simulink subsystem "ButtonStatesRF"  (R2023b) (continued)
== stateflow chart ButtonStatesRF/ButtonStates/buttonFlowChart == (continued)
                  Flow.ButtonTriggeredMenu.Menu.MainSubMenu.SettingsSubMenu.FavoritesSubMenu.Slot1Menu.Slot1FavoritesMenu.Slot1Date -> Flow.ButtonTriggeredMenu.Menu.MainSubMenu.SettingsSubMenu.FavoritesSubMenu.Slot1Menu.Slot1FavoritesMenu.Slot1Battery [order 2] : DOWN
                  Flow.ButtonTriggeredMenu.Menu.MainSubMenu.SettingsSubMenu.FavoritesSubMenu.Slot1Menu.Slot1FavoritesMenu.Slot1Battery -> Flow.ButtonTriggeredMenu.Menu.MainSubMenu.SettingsSubMenu.FavoritesSubMenu.Slot1Menu.Slot1FavoritesMenu.Slot1Date [order 1] : UP
                  Flow.ButtonTriggeredMenu.Menu.MainSubMenu.SettingsSubMenu.FavoritesSubMenu.Slot1Menu.Slot1FavoritesMenu.Slot1Battery -> Flow.ButtonTriggeredMenu.Menu.MainSubMenu.SettingsSubMenu.FavoritesSubMenu.Slot1Menu.Slot1FavoritesMenu.Slot1WaterTemperature [order 2] : DOWN
                  Flow.ButtonTriggeredMenu.Menu.MainSubMenu.SettingsSubMenu.FavoritesSubMenu.Slot1Menu.Slot1FavoritesMenu.Slot1WaterTemperature -> Flow.ButtonTriggeredMenu.Menu.MainSubMenu.SettingsSubMenu.FavoritesSubMenu.Slot1Menu.Slot1FavoritesMenu.Slot1Battery [order 1] : UP
                  Flow.ButtonTriggeredMenu.Menu.MainSubMenu.SettingsSubMenu.FavoritesSubMenu.Slot1Menu.Slot1FavoritesMenu.Slot1WaterTemperature -> Flow.ButtonTriggeredMenu.Menu.MainSubMenu.SettingsSubMenu.FavoritesSubMenu.Slot1Menu.Slot1FavoritesMenu.Slot1Odometer [order 2] : DOWN
                  Flow.ButtonTriggeredMenu.Menu.MainSubMenu.SettingsSubMenu.FavoritesSubMenu.Slot1Menu.Slot1FavoritesMenu.Slot1Odometer -> Flow.ButtonTriggeredMenu.Menu.MainSubMenu.SettingsSubMenu.FavoritesSubMenu.Slot1Menu.Slot1FavoritesMenu.Slot1WaterTemperature [order 1] : UP
                  Flow.ButtonTriggeredMenu.Menu.MainSubMenu.SettingsSubMenu.FavoritesSubMenu.Slot1Menu.Slot1FavoritesMenu.Slot1Odometer -> Flow.ButtonTriggeredMenu.Menu.MainSubMenu.SettingsSubMenu.FavoritesSubMenu.Slot1Menu.Slot1FavoritesMenu.Slot1Connectivity [order 2] : DOWN
                  Flow.ButtonTriggeredMenu.Menu.MainSubMenu.SettingsSubMenu.FavoritesSubMenu.Slot1Menu.Slot1FavoritesMenu.Slot1Connectivity -> Flow.ButtonTriggeredMenu.Menu.MainSubMenu.SettingsSubMenu.FavoritesSubMenu.Slot1Menu.Slot1FavoritesMenu.Slot1Odometer [order 1] : UP
                  (junction 6434) -> Flow.ButtonTriggeredMenu.Menu.MainSubMenu.SettingsSubMenu.FavoritesSubMenu.Slot1Menu.Slot1FavoritesMenu.Slot1Connectivity
                  Flow.ButtonTriggeredMenu.Menu.MainSubMenu.SettingsSubMenu.FavoritesSubMenu.Slot1Menu.Slot1FavoritesMenu.Slot1Connectivity -> (junction 6435) [order 2] : DOWN
                state Slot1FavoritesMenu1 [commented out]
                  state Slot1TripDistance1 [commented out]
                    en:
                    currentState = eStateName.FAVORITES_TRIP_DISTANCE_1;
                  state Slot1AverageSpeed1 [commented out]
                    en:
                    currentState = eStateName.FAVORITES_AVERAGE_SPEED_1;
                  state Slot1AverageFuelCons1 [commented out]
                    en:
                    currentState = eStateName.FAVORITES_AVERAGE_FUEL_CONS_1;
                  state Slot1TripTime1 [commented out]
                    en:
                    currentState = eStateName.FAVORITES_TRIP_TIME_1;
                  state Slot1AverageTripDistance2 [commented out]
                    en:
                    currentState = eStateName.FAVORITES_TRIP_DISTANCE_2;
                  state Slot1AverageSpeed2 [commented out]
                    en:
                    currentState = eStateName.FAVORITES_AVERAGE_SPEED_2;
                  state Slot1AverageFuelCons2 [commented out]
                    en:
                    currentState = eStateName.FAVORITES_AVERAGE_FUEL_CONS_2;
                  state Slot1TripTime2 [commented out]
                    en:
                    currentState = eStateName.FAVORITES_TRIP_TIME_2;
                  state Slot1FuelRange [commented out]
                    en:
                    currentState = eStateName.FAVORITES_FUEL_RANGE;
                  state Slot1Date [commented out]
                    en:
                    currentState = eStateName.FAVORITES_DATE;
                  state Slot1Battery [commented out]
                    en:
                    currentState = eStateName.FAVORITES_BATTERY;
                  state Slot1WaterTemperature [commented out]
                    en:
                    currentState = eStateName.FAVORITES_WATER_TEMPERATURE;
                  state Slot1Odometer [commented out]
                    en:
                    currentState = eStateName.FAVORITES_ODOMETER;
                  state Slot1Connectivity [commented out]
                    en:
                    currentState = eStateName.FAVORITES_CONNECTIVITY;
                  8721 -> (junction 8703) [commented out]
                  (default) -> (junction 8703) [commented out]
                  (junction 8703) -> (junction 8704) [commented out] : [favSlot1Sel ==eStateName.FAVORITES_TRIP_DISTANCE_1 ]
                  (junction 8704) -> Flow.ButtonTriggeredMenu.Menu.MainSubMenu.SettingsSubMenu.FavoritesSubMenu.Slot1Menu.Slot1FavoritesMenu1.Slot1TripDistance1 [commented out]
                  Flow.ButtonTriggeredMenu.Menu.MainSubMenu.SettingsSubMenu.FavoritesSubMenu.Slot1Menu.Slot1FavoritesMenu1.Slot1TripDistance1 -> (junction 8705) [commented out] : UP
                  (junction 8703) -> (junction 8706) [commented out]
                  (junction 8720) -> (junction 8704) [commented out]
                  (junction 8705) -> (junction 8719) [commented out]
                  Flow.ButtonTriggeredMenu.Menu.MainSubMenu.SettingsSubMenu.FavoritesSubMenu.Slot1Menu.Slot1FavoritesMenu1.Slot1TripDistance1 -> Flow.ButtonTriggeredMenu.Menu.MainSubMenu.SettingsSubMenu.FavoritesSubMenu.Slot1Menu.Slot1FavoritesMenu1.Slot1AverageSpeed1 [commented out] : DOWN
                  Flow.ButtonTriggeredMenu.Menu.MainSubMenu.SettingsSubMenu.FavoritesSubMenu.Slot1Menu.Slot1FavoritesMenu1.Slot1AverageSpeed1 -> Flow.ButtonTriggeredMenu.Menu.MainSubMenu.SettingsSubMenu.FavoritesSubMenu.Slot1Menu.Slot1FavoritesMenu1.Slot1TripDistance1 [commented out] : UP
                  (junction 8706) -> Flow.ButtonTriggeredMenu.Menu.MainSubMenu.SettingsSubMenu.FavoritesSubMenu.Slot1Menu.Slot1FavoritesMenu1.Slot1AverageSpeed1 [commented out] : [favSlot1Sel ==eStateName.FAVORITES_AVERAGE_SPEED_1 ]
                  (junction 8706) -> (junction 8707) [commented out]
                  Flow.ButtonTriggeredMenu.Menu.MainSubMenu.SettingsSubMenu.FavoritesSubMenu.Slot1Menu.Slot1FavoritesMenu1.Slot1AverageSpeed1 -> Flow.ButtonTriggeredMenu.Menu.MainSubMenu.SettingsSubMenu.FavoritesSubMenu.Slot1Menu.Slot1FavoritesMenu1.Slot1AverageFuelCons1 [commented out] : DOWN
                  Flow.ButtonTriggeredMenu.Menu.MainSubMenu.SettingsSubMenu.FavoritesSubMenu.Slot1Menu.Slot1FavoritesMenu1.Slot1AverageFuelCons1 -> Flow.ButtonTriggeredMenu.Menu.MainSubMenu.SettingsSubMenu.FavoritesSubMenu.Slot1Menu.Slot1FavoritesMenu1.Slot1AverageSpeed1 [commented out] : UP
                  (junction 8707) -> Flow.ButtonTriggeredMenu.Menu.MainSubMenu.SettingsSubMenu.FavoritesSubMenu.Slot1Menu.Slot1FavoritesMenu1.Slot1AverageFuelCons1 [commented out] : [favSlot1Sel ==eStateName.FAVORITES_AVERAGE_FUEL_CONS_1 ]
                  (junction 8707) -> (junction 8708) [commented out]
                  Flow.ButtonTriggeredMenu.Menu.MainSubMenu.SettingsSubMenu.FavoritesSubMenu.Slot1Menu.Slot1FavoritesMenu1.Slot1AverageFuelCons1 -> Flow.ButtonTriggeredMenu.Menu.MainSubMenu.SettingsSubMenu.FavoritesSubMenu.Slot1Menu.Slot1FavoritesMenu1.Slot1TripTime1 [commented out] : DOWN
                  Flow.ButtonTriggeredMenu.Menu.MainSubMenu.SettingsSubMenu.FavoritesSubMenu.Slot1Menu.Slot1FavoritesMenu1.Slot1TripTime1 -> Flow.ButtonTriggeredMenu.Menu.MainSubMenu.SettingsSubMenu.FavoritesSubMenu.Slot1Menu.Slot1FavoritesMenu1.Slot1AverageFuelCons1 [commented out] : UP
                  (junction 8708) -> Flow.ButtonTriggeredMenu.Menu.MainSubMenu.SettingsSubMenu.FavoritesSubMenu.Slot1Menu.Slot1FavoritesMenu1.Slot1TripTime1 [commented out] : [favSlot1Sel ==eStateName.FAVORITES_TRIP_TIME_1 ]
                  (junction 8708) -> (junction 8709) [commented out]
                  Flow.ButtonTriggeredMenu.Menu.MainSubMenu.SettingsSubMenu.FavoritesSubMenu.Slot1Menu.Slot1FavoritesMenu1.Slot1TripTime1 -> Flow.ButtonTriggeredMenu.Menu.MainSubMenu.SettingsSubMenu.FavoritesSubMenu.Slot1Menu.Slot1FavoritesMenu1.Slot1AverageTripDistance2 [commented out] : DOWN
                  Flow.ButtonTriggeredMenu.Menu.MainSubMenu.SettingsSubMenu.FavoritesSubMenu.Slot1Menu.Slot1FavoritesMenu1.Slot1AverageTripDistance2 -> Flow.ButtonTriggeredMenu.Menu.MainSubMenu.SettingsSubMenu.FavoritesSubMenu.Slot1Menu.Slot1FavoritesMenu1.Slot1TripTime1 [commented out] : UP
                  (junction 8709) -> Flow.ButtonTriggeredMenu.Menu.MainSubMenu.SettingsSubMenu.FavoritesSubMenu.Slot1Menu.Slot1FavoritesMenu1.Slot1AverageTripDistance2 [commented out] : [favSlot1Sel ==eStateName.FAVORITES_TRIP_DISTANCE_2 ]
                  (junction 8709) -> (junction 8710) [commented out]
                  Flow.ButtonTriggeredMenu.Menu.MainSubMenu.SettingsSubMenu.FavoritesSubMenu.Slot1Menu.Slot1FavoritesMenu1.Slot1AverageTripDistance2 -> Flow.ButtonTriggeredMenu.Menu.MainSubMenu.SettingsSubMenu.FavoritesSubMenu.Slot1Menu.Slot1FavoritesMenu1.Slot1AverageSpeed2 [commented out] : DOWN
                  Flow.ButtonTriggeredMenu.Menu.MainSubMenu.SettingsSubMenu.FavoritesSubMenu.Slot1Menu.Slot1FavoritesMenu1.Slot1AverageSpeed2 -> Flow.ButtonTriggeredMenu.Menu.MainSubMenu.SettingsSubMenu.FavoritesSubMenu.Slot1Menu.Slot1FavoritesMenu1.Slot1AverageTripDistance2 [commented out] : UP
                  (junction 8710) -> Flow.ButtonTriggeredMenu.Menu.MainSubMenu.SettingsSubMenu.FavoritesSubMenu.Slot1Menu.Slot1FavoritesMenu1.Slot1AverageSpeed2 [commented out] : [favSlot1Sel ==eStateName.FAVORITES_AVERAGE_SPEED_2 ]
                  (junction 8710) -> (junction 8711) [commented out]
                  Flow.ButtonTriggeredMenu.Menu.MainSubMenu.SettingsSubMenu.FavoritesSubMenu.Slot1Menu.Slot1FavoritesMenu1.Slot1AverageSpeed2 -> Flow.ButtonTriggeredMenu.Menu.MainSubMenu.SettingsSubMenu.FavoritesSubMenu.Slot1Menu.Slot1FavoritesMenu1.Slot1AverageFuelCons2 [commented out] : DOWN
                  Flow.ButtonTriggeredMenu.Menu.MainSubMenu.SettingsSubMenu.FavoritesSubMenu.Slot1Menu.Slot1FavoritesMenu1.Slot1AverageFuelCons2 -> Flow.ButtonTriggeredMenu.Menu.MainSubMenu.SettingsSubMenu.FavoritesSubMenu.Slot1Menu.Slot1FavoritesMenu1.Slot1AverageSpeed2 [commented out] : UP
                  (junction 8711) -> Flow.ButtonTriggeredMenu.Menu.MainSubMenu.SettingsSubMenu.FavoritesSubMenu.Slot1Menu.Slot1FavoritesMenu1.Slot1AverageFuelCons2 [commented out] : [favSlot1Sel ==eStateName.FAVORITES_AVERAGE_FUEL_CONS_2 ]
                  (junction 8711) -> (junction 8712) [commented out]
                  Flow.ButtonTriggeredMenu.Menu.MainSubMenu.SettingsSubMenu.FavoritesSubMenu.Slot1Menu.Slot1FavoritesMenu1.Slot1AverageFuelCons2 -> Flow.ButtonTriggeredMenu.Menu.MainSubMenu.SettingsSubMenu.FavoritesSubMenu.Slot1Menu.Slot1FavoritesMenu1.Slot1TripTime2 [commented out] : DOWN
                  Flow.ButtonTriggeredMenu.Menu.MainSubMenu.SettingsSubMenu.FavoritesSubMenu.Slot1Menu.Slot1FavoritesMenu1.Slot1TripTime2 -> Flow.ButtonTriggeredMenu.Menu.MainSubMenu.SettingsSubMenu.FavoritesSubMenu.Slot1Menu.Slot1FavoritesMenu1.Slot1AverageFuelCons2 [commented out] : UP
                  (junction 8712) -> Flow.ButtonTriggeredMenu.Menu.MainSubMenu.SettingsSubMenu.FavoritesSubMenu.Slot1Menu.Slot1FavoritesMenu1.Slot1TripTime2 [commented out] : [favSlot1Sel ==eStateName.FAVORITES_TRIP_TIME_2 ]
                  (junction 8712) -> (junction 8713) [commented out]
                  Flow.ButtonTriggeredMenu.Menu.MainSubMenu.SettingsSubMenu.FavoritesSubMenu.Slot1Menu.Slot1FavoritesMenu1.Slot1TripTime2 -> Flow.ButtonTriggeredMenu.Menu.MainSubMenu.SettingsSubMenu.FavoritesSubMenu.Slot1Menu.Slot1FavoritesMenu1.Slot1FuelRange [commented out] : DOWN
                  Flow.ButtonTriggeredMenu.Menu.MainSubMenu.SettingsSubMenu.FavoritesSubMenu.Slot1Menu.Slot1FavoritesMenu1.Slot1FuelRange -> Flow.ButtonTriggeredMenu.Menu.MainSubMenu.SettingsSubMenu.FavoritesSubMenu.Slot1Menu.Slot1FavoritesMenu1.Slot1TripTime2 [commented out] : UP
                  (junction 8713) -> Flow.ButtonTriggeredMenu.Menu.MainSubMenu.SettingsSubMenu.FavoritesSubMenu.Slot1Menu.Slot1FavoritesMenu1.Slot1FuelRange [commented out] : [favSlot1Sel ==eStateName.FAVORITES_FUEL_RANGE ]
                  (junction 8713) -> (junction 8714) [commented out]
                  Flow.ButtonTriggeredMenu.Menu.MainSubMenu.SettingsSubMenu.FavoritesSubMenu.Slot1Menu.Slot1FavoritesMenu1.Slot1FuelRange -> Flow.ButtonTriggeredMenu.Menu.MainSubMenu.SettingsSubMenu.FavoritesSubMenu.Slot1Menu.Slot1FavoritesMenu1.Slot1Date [commented out] : DOWN
                  Flow.ButtonTriggeredMenu.Menu.MainSubMenu.SettingsSubMenu.FavoritesSubMenu.Slot1Menu.Slot1FavoritesMenu1.Slot1Date -> Flow.ButtonTriggeredMenu.Menu.MainSubMenu.SettingsSubMenu.FavoritesSubMenu.Slot1Menu.Slot1FavoritesMenu1.Slot1FuelRange [commented out] : UP
                  (junction 8714) -> Flow.ButtonTriggeredMenu.Menu.MainSubMenu.SettingsSubMenu.FavoritesSubMenu.Slot1Menu.Slot1FavoritesMenu1.Slot1Date [commented out] : [favSlot1Sel ==eStateName.FAVORITES_DATE ]
                  (junction 8714) -> (junction 8715) [commented out]
                  Flow.ButtonTriggeredMenu.Menu.MainSubMenu.SettingsSubMenu.FavoritesSubMenu.Slot1Menu.Slot1FavoritesMenu1.Slot1Date -> Flow.ButtonTriggeredMenu.Menu.MainSubMenu.SettingsSubMenu.FavoritesSubMenu.Slot1Menu.Slot1FavoritesMenu1.Slot1Battery [commented out] : DOWN
                  Flow.ButtonTriggeredMenu.Menu.MainSubMenu.SettingsSubMenu.FavoritesSubMenu.Slot1Menu.Slot1FavoritesMenu1.Slot1Battery -> Flow.ButtonTriggeredMenu.Menu.MainSubMenu.SettingsSubMenu.FavoritesSubMenu.Slot1Menu.Slot1FavoritesMenu1.Slot1Date [commented out] : UP
                  (junction 8715) -> Flow.ButtonTriggeredMenu.Menu.MainSubMenu.SettingsSubMenu.FavoritesSubMenu.Slot1Menu.Slot1FavoritesMenu1.Slot1Battery [commented out] : [favSlot1Sel ==eStateName.FAVORITES_BATTERY ]
                  (junction 8715) -> (junction 8716) [commented out]
                  Flow.ButtonTriggeredMenu.Menu.MainSubMenu.SettingsSubMenu.FavoritesSubMenu.Slot1Menu.Slot1FavoritesMenu1.Slot1Battery -> Flow.ButtonTriggeredMenu.Menu.MainSubMenu.SettingsSubMenu.FavoritesSubMenu.Slot1Menu.Slot1FavoritesMenu1.Slot1WaterTemperature [commented out] : DOWN
                  Flow.ButtonTriggeredMenu.Menu.MainSubMenu.SettingsSubMenu.FavoritesSubMenu.Slot1Menu.Slot1FavoritesMenu1.Slot1WaterTemperature -> Flow.ButtonTriggeredMenu.Menu.MainSubMenu.SettingsSubMenu.FavoritesSubMenu.Slot1Menu.Slot1FavoritesMenu1.Slot1Battery [commented out] : UP
                  (junction 8716) -> Flow.ButtonTriggeredMenu.Menu.MainSubMenu.SettingsSubMenu.FavoritesSubMenu.Slot1Menu.Slot1FavoritesMenu1.Slot1WaterTemperature [commented out] : [favSlot1Sel ==eStateName.FAVORITES_WATER_TEMPERATURE ]
                  (junction 8716) -> (junction 8717) [commented out]
                  Flow.ButtonTriggeredMenu.Menu.MainSubMenu.SettingsSubMenu.FavoritesSubMenu.Slot1Menu.Slot1FavoritesMenu1.Slot1WaterTemperature -> Flow.ButtonTriggeredMenu.Menu.MainSubMenu.SettingsSubMenu.FavoritesSubMenu.Slot1Menu.Slot1FavoritesMenu1.Slot1Odometer [commented out] : DOWN
                  Flow.ButtonTriggeredMenu.Menu.MainSubMenu.SettingsSubMenu.FavoritesSubMenu.Slot1Menu.Slot1FavoritesMenu1.Slot1Odometer -> Flow.ButtonTriggeredMenu.Menu.MainSubMenu.SettingsSubMenu.FavoritesSubMenu.Slot1Menu.Slot1FavoritesMenu1.Slot1WaterTemperature [commented out] : UP
                  (junction 8717) -> Flow.ButtonTriggeredMenu.Menu.MainSubMenu.SettingsSubMenu.FavoritesSubMenu.Slot1Menu.Slot1FavoritesMenu1.Slot1Odometer [commented out] : [favSlot1Sel ==eStateName.FAVORITES_ODOMETER ]
                  (junction 8717) -> (junction 8718) [commented out]
                  Flow.ButtonTriggeredMenu.Menu.MainSubMenu.SettingsSubMenu.FavoritesSubMenu.Slot1Menu.Slot1FavoritesMenu1.Slot1Odometer -> Flow.ButtonTriggeredMenu.Menu.MainSubMenu.SettingsSubMenu.FavoritesSubMenu.Slot1Menu.Slot1FavoritesMenu1.Slot1Connectivity [commented out] : DOWN
                  Flow.ButtonTriggeredMenu.Menu.MainSubMenu.SettingsSubMenu.FavoritesSubMenu.Slot1Menu.Slot1FavoritesMenu1.Slot1Connectivity -> Flow.ButtonTriggeredMenu.Menu.MainSubMenu.SettingsSubMenu.FavoritesSubMenu.Slot1Menu.Slot1FavoritesMenu1.Slot1Odometer [commented out] : UP
                  (junction 8718) -> Flow.ButtonTriggeredMenu.Menu.MainSubMenu.SettingsSubMenu.FavoritesSubMenu.Slot1Menu.Slot1FavoritesMenu1.Slot1Connectivity [commented out]
                  (junction 8719) -> Flow.ButtonTriggeredMenu.Menu.MainSubMenu.SettingsSubMenu.FavoritesSubMenu.Slot1Menu.Slot1FavoritesMenu1.Slot1Connectivity [commented out]
                  Flow.ButtonTriggeredMenu.Menu.MainSubMenu.SettingsSubMenu.FavoritesSubMenu.Slot1Menu.Slot1FavoritesMenu1.Slot1Connectivity -> (junction 8720) [commented out] : DOWN
                state favSlot1Updater(currState) [function]
                  (default) -> (junction 6485) : %{tempSlotSel  = eStateName.FAVORITES_TRIP_DISTANCE_1;}
                  (junction 6485) -> (junction 6486) [order 1] : [currState == favSlot2Sel ]
                  (junction 6486) -> (junction 6487) : { favSlot2Sel = favSlot1Sel;
                      | BLU_FnSaveSlot2Fav(favSlot2Sel);}
                  (junction 6485) -> (junction 6488) [order 2]
                  (junction 6487) -> (junction 6490)
                  (junction 6488) -> (junction 6489) [order 1] : [currState == favSlot3Sel]
                  (junction 6489) -> (junction 6490) : {favSlot3Sel = favSlot1Sel;
                      | BLU_FnSaveSlot3Fav(favSlot3Sel);}
                  (junction 6488) -> (junction 6491) [order 2]
                  (junction 6490) -> (junction 6493)
                  (junction 6491) -> (junction 6492) [order 1] : [currState == favSlot4Sel ]
                  (junction 6492) -> (junction 6493) : {favSlot4Sel = favSlot1Sel;
                      | BLU_FnSaveSlot4Fav(favSlot4Sel);}
                  (junction 6491) -> (junction 6494) [order 2]
                  (junction 6493) -> (junction 6495)
                  (junction 6495) -> (junction 6494)
                  (junction 6494) -> (junction 6496) : {favSlot1Sel = currState;
                      | BLU_FnSaveSlot1Fav(favSlot1Sel);}
                state favSlotUpdater(currState) [function] [commented out]
                  (default) -> (junction 8448) [commented out] : %{tempSlotSel  = eStateName.FAVORITES_TRIP_DISTANCE_1;}
                  (junction 8448) -> (junction 8449) [commented out] : [currState == favSlot2Sel ]
                  (junction 8449) -> (junction 8450) [commented out] : { favSlot2Sel = favSlot1Sel;
                      | BLU_FnSaveSlot2Fav(favSlot2Sel);}
                  (junction 8448) -> (junction 8451) [commented out]
                  (junction 8450) -> (junction 8453) [commented out]
                  (junction 8451) -> (junction 8452) [commented out] : [currState == favSlot3Sel]
                  (junction 8452) -> (junction 8453) [commented out] : {favSlot3Sel = favSlot1Sel;
                      | BLU_FnSaveSlot3Fav(favSlot3Sel);}
                  (junction 8451) -> (junction 8454) [commented out]
                  (junction 8453) -> (junction 8456) [commented out]
                  (junction 8454) -> (junction 8455) [commented out] : [currState == favSlot4Sel ]
                  (junction 8455) -> (junction 8456) [commented out] : {favSlot4Sel = favSlot1Sel;
                      | BLU_FnSaveSlot4Fav(favSlot4Sel);}
                  (junction 8454) -> (junction 8457) [commented out]
                  (junction 8456) -> (junction 8458) [commented out]
                  (junction 8458) -> (junction 8457) [commented out]
                  (junction 8457) -> (junction 8459) [commented out] : {favSlot1Sel = currState;
                      | BLU_FnSaveSlot1Fav(favSlot1Sel);}
                6424 -> Slot1FavoritesMenu
                Slot1FavoritesMenu -> 6425 [order 2] : EXIT
                (junction 6423) -> 6425 : {favSlot1Updater(currentState);}
                Slot1FavoritesMenu -> (junction 6423) [order 1] : SET
              state Slot2Menu
                state Slot2FavoritesMenu
                  (history)
                  state Slot2TripDistance1
                    en:
                    currentState = eStateName.FAVORITES_TRIP_DISTANCE_1;
                  state Slot2AverageSpeed1
                    en:
                    currentState = eStateName.FAVORITES_AVERAGE_SPEED_1;
                  state Slot2AverageFuelCons1
                    en:
                    currentState = eStateName.FAVORITES_AVERAGE_FUEL_CONS_1;
                  state Slot2TripTime1
                    en:
                    currentState = eStateName.FAVORITES_TRIP_TIME_1;
                  state Slot2AverageTripDistance2
                    en:
                    currentState = eStateName.FAVORITES_TRIP_DISTANCE_2;
                  state Slot2AverageSpeed2
                    en:
                    currentState = eStateName.FAVORITES_AVERAGE_SPEED_2;
                  state Slot2AverageFuelCons2
                    en:
                    currentState = eStateName.FAVORITES_AVERAGE_FUEL_CONS_2;
                  state Slot2TripTime2
                    en:
                    currentState = eStateName.FAVORITES_TRIP_TIME_2;
                  state Slot2FuelRange
                    en:
                    currentState = eStateName.FAVORITES_FUEL_RANGE;
                  state Slot2Date
                    en:
                    currentState = eStateName.FAVORITES_DATE;
                  state Slot2Battery
                    en:
                    currentState = eStateName.FAVORITES_BATTERY;
                  state Slot2WaterTemperature
                    en:
                    currentState = eStateName.FAVORITES_WATER_TEMPERATURE;
                  state Slot2Odometer
                    en:
                    currentState = eStateName.FAVORITES_ODOMETER;
                  state Slot2Connectivity
                    en:
                    currentState = eStateName.FAVORITES_CONNECTIVITY;
                  (default) -> (junction 6523)
                  Flow.ButtonTriggeredMenu.Menu.MainSubMenu.SettingsSubMenu.FavoritesSubMenu.Slot2Menu.Slot2FavoritesMenu.Slot2TripDistance1 -> (junction 6522) [order 2] : UP
                  (junction 6523) -> Flow.ButtonTriggeredMenu.Menu.MainSubMenu.SettingsSubMenu.FavoritesSubMenu.Slot2Menu.Slot2FavoritesMenu.Slot2TripDistance1
                  (junction 6522) -> (junction 6525)
                  (junction 6524) -> (junction 6523)
                  Flow.ButtonTriggeredMenu.Menu.MainSubMenu.SettingsSubMenu.FavoritesSubMenu.Slot2Menu.Slot2FavoritesMenu.Slot2TripDistance1 -> Flow.ButtonTriggeredMenu.Menu.MainSubMenu.SettingsSubMenu.FavoritesSubMenu.Slot2Menu.Slot2FavoritesMenu.Slot2AverageSpeed1 [order 1] : DOWN
                  Flow.ButtonTriggeredMenu.Menu.MainSubMenu.SettingsSubMenu.FavoritesSubMenu.Slot2Menu.Slot2FavoritesMenu.Slot2AverageSpeed1 -> Flow.ButtonTriggeredMenu.Menu.MainSubMenu.SettingsSubMenu.FavoritesSubMenu.Slot2Menu.Slot2FavoritesMenu.Slot2TripDistance1 [order 1] : UP
                  Flow.ButtonTriggeredMenu.Menu.MainSubMenu.SettingsSubMenu.FavoritesSubMenu.Slot2Menu.Slot2FavoritesMenu.Slot2AverageSpeed1 -> Flow.ButtonTriggeredMenu.Menu.MainSubMenu.SettingsSubMenu.FavoritesSubMenu.Slot2Menu.Slot2FavoritesMenu.Slot2AverageFuelCons1 [order 2] : DOWN
                  Flow.ButtonTriggeredMenu.Menu.MainSubMenu.SettingsSubMenu.FavoritesSubMenu.Slot2Menu.Slot2FavoritesMenu.Slot2AverageFuelCons1 -> Flow.ButtonTriggeredMenu.Menu.MainSubMenu.SettingsSubMenu.FavoritesSubMenu.Slot2Menu.Slot2FavoritesMenu.Slot2AverageSpeed1 [order 1] : UP
                  Flow.ButtonTriggeredMenu.Menu.MainSubMenu.SettingsSubMenu.FavoritesSubMenu.Slot2Menu.Slot2FavoritesMenu.Slot2AverageFuelCons1 -> Flow.ButtonTriggeredMenu.Menu.MainSubMenu.SettingsSubMenu.FavoritesSubMenu.Slot2Menu.Slot2FavoritesMenu.Slot2TripTime1 [order 2] : DOWN
                  Flow.ButtonTriggeredMenu.Menu.MainSubMenu.SettingsSubMenu.FavoritesSubMenu.Slot2Menu.Slot2FavoritesMenu.Slot2TripTime1 -> Flow.ButtonTriggeredMenu.Menu.MainSubMenu.SettingsSubMenu.FavoritesSubMenu.Slot2Menu.Slot2FavoritesMenu.Slot2AverageFuelCons1 [order 1] : UP
                  Flow.ButtonTriggeredMenu.Menu.MainSubMenu.SettingsSubMenu.FavoritesSubMenu.Slot2Menu.Slot2FavoritesMenu.Slot2TripTime1 -> Flow.ButtonTriggeredMenu.Menu.MainSubMenu.SettingsSubMenu.FavoritesSubMenu.Slot2Menu.Slot2FavoritesMenu.Slot2AverageTripDistance2 [order 2] : DOWN
                  Flow.ButtonTriggeredMenu.Menu.MainSubMenu.SettingsSubMenu.FavoritesSubMenu.Slot2Menu.Slot2FavoritesMenu.Slot2AverageTripDistance2 -> Flow.ButtonTriggeredMenu.Menu.MainSubMenu.SettingsSubMenu.FavoritesSubMenu.Slot2Menu.Slot2FavoritesMenu.Slot2TripTime1 [order 1] : UP
                  Flow.ButtonTriggeredMenu.Menu.MainSubMenu.SettingsSubMenu.FavoritesSubMenu.Slot2Menu.Slot2FavoritesMenu.Slot2AverageTripDistance2 -> Flow.ButtonTriggeredMenu.Menu.MainSubMenu.SettingsSubMenu.FavoritesSubMenu.Slot2Menu.Slot2FavoritesMenu.Slot2AverageSpeed2 [order 2] : DOWN
                  Flow.ButtonTriggeredMenu.Menu.MainSubMenu.SettingsSubMenu.FavoritesSubMenu.Slot2Menu.Slot2FavoritesMenu.Slot2AverageSpeed2 -> Flow.ButtonTriggeredMenu.Menu.MainSubMenu.SettingsSubMenu.FavoritesSubMenu.Slot2Menu.Slot2FavoritesMenu.Slot2AverageTripDistance2 [order 1] : UP
                  Flow.ButtonTriggeredMenu.Menu.MainSubMenu.SettingsSubMenu.FavoritesSubMenu.Slot2Menu.Slot2FavoritesMenu.Slot2AverageSpeed2 -> Flow.ButtonTriggeredMenu.Menu.MainSubMenu.SettingsSubMenu.FavoritesSubMenu.Slot2Menu.Slot2FavoritesMenu.Slot2AverageFuelCons2 [order 2] : DOWN
                  Flow.ButtonTriggeredMenu.Menu.MainSubMenu.SettingsSubMenu.FavoritesSubMenu.Slot2Menu.Slot2FavoritesMenu.Slot2AverageFuelCons2 -> Flow.ButtonTriggeredMenu.Menu.MainSubMenu.SettingsSubMenu.FavoritesSubMenu.Slot2Menu.Slot2FavoritesMenu.Slot2AverageSpeed2 [order 1] : UP
                  Flow.ButtonTriggeredMenu.Menu.MainSubMenu.SettingsSubMenu.FavoritesSubMenu.Slot2Menu.Slot2FavoritesMenu.Slot2AverageFuelCons2 -> Flow.ButtonTriggeredMenu.Menu.MainSubMenu.SettingsSubMenu.FavoritesSubMenu.Slot2Menu.Slot2FavoritesMenu.Slot2TripTime2 [order 2] : DOWN
                  Flow.ButtonTriggeredMenu.Menu.MainSubMenu.SettingsSubMenu.FavoritesSubMenu.Slot2Menu.Slot2FavoritesMenu.Slot2TripTime2 -> Flow.ButtonTriggeredMenu.Menu.MainSubMenu.SettingsSubMenu.FavoritesSubMenu.Slot2Menu.Slot2FavoritesMenu.Slot2AverageFuelCons2 [order 1] : UP
                  Flow.ButtonTriggeredMenu.Menu.MainSubMenu.SettingsSubMenu.FavoritesSubMenu.Slot2Menu.Slot2FavoritesMenu.Slot2TripTime2 -> Flow.ButtonTriggeredMenu.Menu.MainSubMenu.SettingsSubMenu.FavoritesSubMenu.Slot2Menu.Slot2FavoritesMenu.Slot2FuelRange [order 2] : DOWN
                  Flow.ButtonTriggeredMenu.Menu.MainSubMenu.SettingsSubMenu.FavoritesSubMenu.Slot2Menu.Slot2FavoritesMenu.Slot2FuelRange -> Flow.ButtonTriggeredMenu.Menu.MainSubMenu.SettingsSubMenu.FavoritesSubMenu.Slot2Menu.Slot2FavoritesMenu.Slot2TripTime2 [order 1] : UP
                  Flow.ButtonTriggeredMenu.Menu.MainSubMenu.SettingsSubMenu.FavoritesSubMenu.Slot2Menu.Slot2FavoritesMenu.Slot2FuelRange -> Flow.ButtonTriggeredMenu.Menu.MainSubMenu.SettingsSubMenu.FavoritesSubMenu.Slot2Menu.Slot2FavoritesMenu.Slot2Date [order 2] : DOWN
                  Flow.ButtonTriggeredMenu.Menu.MainSubMenu.SettingsSubMenu.FavoritesSubMenu.Slot2Menu.Slot2FavoritesMenu.Slot2Date -> Flow.ButtonTriggeredMenu.Menu.MainSubMenu.SettingsSubMenu.FavoritesSubMenu.Slot2Menu.Slot2FavoritesMenu.Slot2FuelRange [order 1] : UP
                  Flow.ButtonTriggeredMenu.Menu.MainSubMenu.SettingsSubMenu.FavoritesSubMenu.Slot2Menu.Slot2FavoritesMenu.Slot2Date -> Flow.ButtonTriggeredMenu.Menu.MainSubMenu.SettingsSubMenu.FavoritesSubMenu.Slot2Menu.Slot2FavoritesMenu.Slot2Battery [order 2] : DOWN
                  Flow.ButtonTriggeredMenu.Menu.MainSubMenu.SettingsSubMenu.FavoritesSubMenu.Slot2Menu.Slot2FavoritesMenu.Slot2Battery -> Flow.ButtonTriggeredMenu.Menu.MainSubMenu.SettingsSubMenu.FavoritesSubMenu.Slot2Menu.Slot2FavoritesMenu.Slot2Date [order 1] : UP
                  Flow.ButtonTriggeredMenu.Menu.MainSubMenu.SettingsSubMenu.FavoritesSubMenu.Slot2Menu.Slot2FavoritesMenu.Slot2Battery -> Flow.ButtonTriggeredMenu.Menu.MainSubMenu.SettingsSubMenu.FavoritesSubMenu.Slot2Menu.Slot2FavoritesMenu.Slot2WaterTemperature [order 2] : DOWN
                  Flow.ButtonTriggeredMenu.Menu.MainSubMenu.SettingsSubMenu.FavoritesSubMenu.Slot2Menu.Slot2FavoritesMenu.Slot2WaterTemperature -> Flow.ButtonTriggeredMenu.Menu.MainSubMenu.SettingsSubMenu.FavoritesSubMenu.Slot2Menu.Slot2FavoritesMenu.Slot2Battery [order 1] : UP
                  Flow.ButtonTriggeredMenu.Menu.MainSubMenu.SettingsSubMenu.FavoritesSubMenu.Slot2Menu.Slot2FavoritesMenu.Slot2WaterTemperature -> Flow.ButtonTriggeredMenu.Menu.MainSubMenu.SettingsSubMenu.FavoritesSubMenu.Slot2Menu.Slot2FavoritesMenu.Slot2Odometer [order 2] : DOWN
                  Flow.ButtonTriggeredMenu.Menu.MainSubMenu.SettingsSubMenu.FavoritesSubMenu.Slot2Menu.Slot2FavoritesMenu.Slot2Odometer -> Flow.ButtonTriggeredMenu.Menu.MainSubMenu.SettingsSubMenu.FavoritesSubMenu.Slot2Menu.Slot2FavoritesMenu.Slot2WaterTemperature [order 1] : UP
                  Flow.ButtonTriggeredMenu.Menu.MainSubMenu.SettingsSubMenu.FavoritesSubMenu.Slot2Menu.Slot2FavoritesMenu.Slot2Odometer -> Flow.ButtonTriggeredMenu.Menu.MainSubMenu.SettingsSubMenu.FavoritesSubMenu.Slot2Menu.Slot2FavoritesMenu.Slot2Connectivity [order 2] : DOWN
                  Flow.ButtonTriggeredMenu.Menu.MainSubMenu.SettingsSubMenu.FavoritesSubMenu.Slot2Menu.Slot2FavoritesMenu.Slot2Connectivity -> Flow.ButtonTriggeredMenu.Menu.MainSubMenu.SettingsSubMenu.FavoritesSubMenu.Slot2Menu.Slot2FavoritesMenu.Slot2Odometer [order 1] : UP
                  Flow.ButtonTriggeredMenu.Menu.MainSubMenu.SettingsSubMenu.FavoritesSubMenu.Slot2Menu.Slot2FavoritesMenu.Slot2Connectivity -> (junction 6524) [order 2] : DOWN
                  (junction 6525) -> Flow.ButtonTriggeredMenu.Menu.MainSubMenu.SettingsSubMenu.FavoritesSubMenu.Slot2Menu.Slot2FavoritesMenu.Slot2Connectivity
                state favSlot2Updater(currState) [function]
                  (default) -> (junction 6575) : %{tempSlotSel  = eStateName.FAVORITES_TRIP_DISTANCE_1;}
                  (junction 6575) -> (junction 6576) [order 1] : [currState == favSlot1Sel ]
                  (junction 6576) -> (junction 6577) : { favSlot1Sel = favSlot2Sel;
                      | BLU_FnSaveSlot1Fav(favSlot1Sel);}
                  (junction 6575) -> (junction 6578) [order 2]
                  (junction 6577) -> (junction 6580)
                  (junction 6578) -> (junction 6579) [order 1] : [currState == favSlot3Sel]
                  (junction 6579) -> (junction 6580) : {favSlot3Sel = favSlot2Sel;
                      | BLU_FnSaveSlot3Fav(favSlot3Sel);}
                  (junction 6578) -> (junction 6581) [order 2]
                  (junction 6580) -> (junction 6583)
                  (junction 6581) -> (junction 6582) [order 1] : [currState == favSlot4Sel ]
                  (junction 6582) -> (junction 6583) : {favSlot4Sel = favSlot2Sel;
                      | BLU_FnSaveSlot4Fav(favSlot4Sel);}
                  (junction 6581) -> (junction 6584) [order 2]
                  (junction 6583) -> (junction 6585)
                  (junction 6585) -> (junction 6584)
                  (junction 6584) -> (junction 6586) : {favSlot2Sel = currState;
                      | BLU_FnSaveSlot2Fav(favSlot2Sel);}
                state Slot2FavoritesMenu1 [commented out]
                  state Slot2TripDistance1 [commented out]
                    en:
                    currentState = eStateName.FAVORITES_TRIP_DISTANCE_1;
                  state Slot2AverageSpeed1 [commented out]
                    en:
                    currentState = eStateName.FAVORITES_AVERAGE_SPEED_1;
                  state Slot2AverageFuelCons1 [commented out]
                    en:
                    currentState = eStateName.FAVORITES_AVERAGE_FUEL_CONS_1;
                  state Slot2TripTime1 [commented out]
                    en:
                    currentState = eStateName.FAVORITES_TRIP_TIME_1;
                  state Slot2AverageTripDistance2 [commented out]
                    en:
                    currentState = eStateName.FAVORITES_TRIP_DISTANCE_2;
                  state Slot2AverageSpeed2 [commented out]
                    en:
                    currentState = eStateName.FAVORITES_AVERAGE_SPEED_2;
                  state Slot2AverageFuelCons2 [commented out]
                    en:
                    currentState = eStateName.FAVORITES_AVERAGE_FUEL_CONS_2;
                  state Slot2TripTime2 [commented out]
                    en:
                    currentState = eStateName.FAVORITES_TRIP_TIME_2;
                  state Slot2FuelRange [commented out]
                    en:
                    currentState = eStateName.FAVORITES_FUEL_RANGE;
                  state Slot2Date [commented out]
                    en:
                    currentState = eStateName.FAVORITES_DATE;
                  state Slot2Battery [commented out]
                    en:
                    currentState = eStateName.FAVORITES_BATTERY;
                  state Slot2WaterTemperature [commented out]
                    en:
                    currentState = eStateName.FAVORITES_WATER_TEMPERATURE;
                  state Slot2Odometer [commented out]
                    en:
                    currentState = eStateName.FAVORITES_ODOMETER;
                  state Slot2Connectivity [commented out]
                    en:
                    currentState = eStateName.FAVORITES_CONNECTIVITY;
                  (default) -> (junction 8803) [commented out]
                  Flow.ButtonTriggeredMenu.Menu.MainSubMenu.SettingsSubMenu.FavoritesSubMenu.Slot2Menu.Slot2FavoritesMenu1.Slot2TripDistance1 -> (junction 8802) [commented out] : UP
                  (junction 8803) -> (junction 8804) [commented out] : [favSlot2Sel ==eStateName.FAVORITES_TRIP_DISTANCE_1 ]
                  (junction 8804) -> Flow.ButtonTriggeredMenu.Menu.MainSubMenu.SettingsSubMenu.FavoritesSubMenu.Slot2Menu.Slot2FavoritesMenu1.Slot2TripDistance1 [commented out]
                  (junction 8802) -> (junction 8818) [commented out]
                  (junction 8803) -> (junction 8805) [commented out]
                  (junction 8817) -> (junction 8804) [commented out]
                  Flow.ButtonTriggeredMenu.Menu.MainSubMenu.SettingsSubMenu.FavoritesSubMenu.Slot2Menu.Slot2FavoritesMenu1.Slot2TripDistance1 -> Flow.ButtonTriggeredMenu.Menu.MainSubMenu.SettingsSubMenu.FavoritesSubMenu.Slot2Menu.Slot2FavoritesMenu1.Slot2AverageSpeed1 [commented out] : DOWN
                  Flow.ButtonTriggeredMenu.Menu.MainSubMenu.SettingsSubMenu.FavoritesSubMenu.Slot2Menu.Slot2FavoritesMenu1.Slot2AverageSpeed1 -> Flow.ButtonTriggeredMenu.Menu.MainSubMenu.SettingsSubMenu.FavoritesSubMenu.Slot2Menu.Slot2FavoritesMenu1.Slot2TripDistance1 [commented out] : UP
                  (junction 8805) -> Flow.ButtonTriggeredMenu.Menu.MainSubMenu.SettingsSubMenu.FavoritesSubMenu.Slot2Menu.Slot2FavoritesMenu1.Slot2AverageSpeed1 [commented out] : [favSlot2Sel ==eStateName.FAVORITES_AVERAGE_SPEED_1 ]
                  (junction 8805) -> (junction 8806) [commented out]
                  Flow.ButtonTriggeredMenu.Menu.MainSubMenu.SettingsSubMenu.FavoritesSubMenu.Slot2Menu.Slot2FavoritesMenu1.Slot2AverageSpeed1 -> Flow.ButtonTriggeredMenu.Menu.MainSubMenu.SettingsSubMenu.FavoritesSubMenu.Slot2Menu.Slot2FavoritesMenu1.Slot2AverageFuelCons1 [commented out] : DOWN
                  Flow.ButtonTriggeredMenu.Menu.MainSubMenu.SettingsSubMenu.FavoritesSubMenu.Slot2Menu.Slot2FavoritesMenu1.Slot2AverageFuelCons1 -> Flow.ButtonTriggeredMenu.Menu.MainSubMenu.SettingsSubMenu.FavoritesSubMenu.Slot2Menu.Slot2FavoritesMenu1.Slot2AverageSpeed1 [commented out] : UP
                  (junction 8806) -> Flow.ButtonTriggeredMenu.Menu.MainSubMenu.SettingsSubMenu.FavoritesSubMenu.Slot2Menu.Slot2FavoritesMenu1.Slot2AverageFuelCons1 [commented out] : [favSlot2Sel ==eStateName.FAVORITES_AVERAGE_FUEL_CONS_1 ]
                  (junction 8806) -> (junction 8807) [commented out]
                  Flow.ButtonTriggeredMenu.Menu.MainSubMenu.SettingsSubMenu.FavoritesSubMenu.Slot2Menu.Slot2FavoritesMenu1.Slot2AverageFuelCons1 -> Flow.ButtonTriggeredMenu.Menu.MainSubMenu.SettingsSubMenu.FavoritesSubMenu.Slot2Menu.Slot2FavoritesMenu1.Slot2TripTime1 [commented out] : DOWN
                  Flow.ButtonTriggeredMenu.Menu.MainSubMenu.SettingsSubMenu.FavoritesSubMenu.Slot2Menu.Slot2FavoritesMenu1.Slot2TripTime1 -> Flow.ButtonTriggeredMenu.Menu.MainSubMenu.SettingsSubMenu.FavoritesSubMenu.Slot2Menu.Slot2FavoritesMenu1.Slot2AverageFuelCons1 [commented out] : UP
                  (junction 8807) -> Flow.ButtonTriggeredMenu.Menu.MainSubMenu.SettingsSubMenu.FavoritesSubMenu.Slot2Menu.Slot2FavoritesMenu1.Slot2TripTime1 [commented out] : [favSlot2Sel ==eStateName.FAVORITES_TRIP_TIME_1 ]
                  (junction 8807) -> (junction 8808) [commented out]
                  Flow.ButtonTriggeredMenu.Menu.MainSubMenu.SettingsSubMenu.FavoritesSubMenu.Slot2Menu.Slot2FavoritesMenu1.Slot2TripTime1 -> Flow.ButtonTriggeredMenu.Menu.MainSubMenu.SettingsSubMenu.FavoritesSubMenu.Slot2Menu.Slot2FavoritesMenu1.Slot2AverageTripDistance2 [commented out] : DOWN
                  Flow.ButtonTriggeredMenu.Menu.MainSubMenu.SettingsSubMenu.FavoritesSubMenu.Slot2Menu.Slot2FavoritesMenu1.Slot2AverageTripDistance2 -> Flow.ButtonTriggeredMenu.Menu.MainSubMenu.SettingsSubMenu.FavoritesSubMenu.Slot2Menu.Slot2FavoritesMenu1.Slot2TripTime1 [commented out] : UP
                  (junction 8808) -> Flow.ButtonTriggeredMenu.Menu.MainSubMenu.SettingsSubMenu.FavoritesSubMenu.Slot2Menu.Slot2FavoritesMenu1.Slot2AverageTripDistance2 [commented out] : [favSlot2Sel ==eStateName.FAVORITES_TRIP_DISTANCE_2 ]
                  (junction 8808) -> (junction 8809) [commented out]
                  Flow.ButtonTriggeredMenu.Menu.MainSubMenu.SettingsSubMenu.FavoritesSubMenu.Slot2Menu.Slot2FavoritesMenu1.Slot2AverageTripDistance2 -> Flow.ButtonTriggeredMenu.Menu.MainSubMenu.SettingsSubMenu.FavoritesSubMenu.Slot2Menu.Slot2FavoritesMenu1.Slot2AverageSpeed2 [commented out] : DOWN
                  Flow.ButtonTriggeredMenu.Menu.MainSubMenu.SettingsSubMenu.FavoritesSubMenu.Slot2Menu.Slot2FavoritesMenu1.Slot2AverageSpeed2 -> Flow.ButtonTriggeredMenu.Menu.MainSubMenu.SettingsSubMenu.FavoritesSubMenu.Slot2Menu.Slot2FavoritesMenu1.Slot2AverageTripDistance2 [commented out] : UP
                  (junction 8809) -> Flow.ButtonTriggeredMenu.Menu.MainSubMenu.SettingsSubMenu.FavoritesSubMenu.Slot2Menu.Slot2FavoritesMenu1.Slot2AverageSpeed2 [commented out] : [favSlot2Sel ==eStateName.FAVORITES_AVERAGE_SPEED_2 ]
                  (junction 8809) -> (junction 8810) [commented out]
                  Flow.ButtonTriggeredMenu.Menu.MainSubMenu.SettingsSubMenu.FavoritesSubMenu.Slot2Menu.Slot2FavoritesMenu1.Slot2AverageSpeed2 -> Flow.ButtonTriggeredMenu.Menu.MainSubMenu.SettingsSubMenu.FavoritesSubMenu.Slot2Menu.Slot2FavoritesMenu1.Slot2AverageFuelCons2 [commented out] : DOWN
                  Flow.ButtonTriggeredMenu.Menu.MainSubMenu.SettingsSubMenu.FavoritesSubMenu.Slot2Menu.Slot2FavoritesMenu1.Slot2AverageFuelCons2 -> Flow.ButtonTriggeredMenu.Menu.MainSubMenu.SettingsSubMenu.FavoritesSubMenu.Slot2Menu.Slot2FavoritesMenu1.Slot2AverageSpeed2 [commented out] : UP
                  (junction 8810) -> Flow.ButtonTriggeredMenu.Menu.MainSubMenu.SettingsSubMenu.FavoritesSubMenu.Slot2Menu.Slot2FavoritesMenu1.Slot2AverageFuelCons2 [commented out] : [favSlot2Sel ==eStateName.FAVORITES_AVERAGE_FUEL_CONS_2 ]
                  (junction 8810) -> (junction 8811) [commented out]
                  Flow.ButtonTriggeredMenu.Menu.MainSubMenu.SettingsSubMenu.FavoritesSubMenu.Slot2Menu.Slot2FavoritesMenu1.Slot2AverageFuelCons2 -> Flow.ButtonTriggeredMenu.Menu.MainSubMenu.SettingsSubMenu.FavoritesSubMenu.Slot2Menu.Slot2FavoritesMenu1.Slot2TripTime2 [commented out] : DOWN
                  Flow.ButtonTriggeredMenu.Menu.MainSubMenu.SettingsSubMenu.FavoritesSubMenu.Slot2Menu.Slot2FavoritesMenu1.Slot2TripTime2 -> Flow.ButtonTriggeredMenu.Menu.MainSubMenu.SettingsSubMenu.FavoritesSubMenu.Slot2Menu.Slot2FavoritesMenu1.Slot2AverageFuelCons2 [commented out] : UP
                  (junction 8811) -> Flow.ButtonTriggeredMenu.Menu.MainSubMenu.SettingsSubMenu.FavoritesSubMenu.Slot2Menu.Slot2FavoritesMenu1.Slot2TripTime2 [commented out] : [favSlot2Sel ==eStateName.FAVORITES_TRIP_TIME_2 ]
                  (junction 8811) -> (junction 8812) [commented out]
                  Flow.ButtonTriggeredMenu.Menu.MainSubMenu.SettingsSubMenu.FavoritesSubMenu.Slot2Menu.Slot2FavoritesMenu1.Slot2TripTime2 -> Flow.ButtonTriggeredMenu.Menu.MainSubMenu.SettingsSubMenu.FavoritesSubMenu.Slot2Menu.Slot2FavoritesMenu1.Slot2FuelRange [commented out] : DOWN
                  Flow.ButtonTriggeredMenu.Menu.MainSubMenu.SettingsSubMenu.FavoritesSubMenu.Slot2Menu.Slot2FavoritesMenu1.Slot2FuelRange -> Flow.ButtonTriggeredMenu.Menu.MainSubMenu.SettingsSubMenu.FavoritesSubMenu.Slot2Menu.Slot2FavoritesMenu1.Slot2TripTime2 [commented out] : UP
                  (junction 8812) -> Flow.ButtonTriggeredMenu.Menu.MainSubMenu.SettingsSubMenu.FavoritesSubMenu.Slot2Menu.Slot2FavoritesMenu1.Slot2FuelRange [commented out] : [favSlot2Sel ==eStateName.FAVORITES_FUEL_RANGE ]
                  (junction 8812) -> (junction 8813) [commented out]
                  Flow.ButtonTriggeredMenu.Menu.MainSubMenu.SettingsSubMenu.FavoritesSubMenu.Slot2Menu.Slot2FavoritesMenu1.Slot2FuelRange -> Flow.ButtonTriggeredMenu.Menu.MainSubMenu.SettingsSubMenu.FavoritesSubMenu.Slot2Menu.Slot2FavoritesMenu1.Slot2Date [commented out] : DOWN
                  Flow.ButtonTriggeredMenu.Menu.MainSubMenu.SettingsSubMenu.FavoritesSubMenu.Slot2Menu.Slot2FavoritesMenu1.Slot2Date -> Flow.ButtonTriggeredMenu.Menu.MainSubMenu.SettingsSubMenu.FavoritesSubMenu.Slot2Menu.Slot2FavoritesMenu1.Slot2FuelRange [commented out] : UP
                  (junction 8813) -> Flow.ButtonTriggeredMenu.Menu.MainSubMenu.SettingsSubMenu.FavoritesSubMenu.Slot2Menu.Slot2FavoritesMenu1.Slot2Date [commented out] : [favSlot2Sel ==eStateName.FAVORITES_DATE ]
                  (junction 8813) -> (junction 8814) [commented out]
                  Flow.ButtonTriggeredMenu.Menu.MainSubMenu.SettingsSubMenu.FavoritesSubMenu.Slot2Menu.Slot2FavoritesMenu1.Slot2Date -> Flow.ButtonTriggeredMenu.Menu.MainSubMenu.SettingsSubMenu.FavoritesSubMenu.Slot2Menu.Slot2FavoritesMenu1.Slot2Battery [commented out] : DOWN
                  Flow.ButtonTriggeredMenu.Menu.MainSubMenu.SettingsSubMenu.FavoritesSubMenu.Slot2Menu.Slot2FavoritesMenu1.Slot2Battery -> Flow.ButtonTriggeredMenu.Menu.MainSubMenu.SettingsSubMenu.FavoritesSubMenu.Slot2Menu.Slot2FavoritesMenu1.Slot2Date [commented out] : UP
                  (junction 8814) -> Flow.ButtonTriggeredMenu.Menu.MainSubMenu.SettingsSubMenu.FavoritesSubMenu.Slot2Menu.Slot2FavoritesMenu1.Slot2Battery [commented out] : [favSlot2Sel ==eStateName.FAVORITES_BATTERY ]
                  (junction 8814) -> (junction 8815) [commented out]
                  Flow.ButtonTriggeredMenu.Menu.MainSubMenu.SettingsSubMenu.FavoritesSubMenu.Slot2Menu.Slot2FavoritesMenu1.Slot2Battery -> Flow.ButtonTriggeredMenu.Menu.MainSubMenu.SettingsSubMenu.FavoritesSubMenu.Slot2Menu.Slot2FavoritesMenu1.Slot2WaterTemperature [commented out] : DOWN
                  Flow.ButtonTriggeredMenu.Menu.MainSubMenu.SettingsSubMenu.FavoritesSubMenu.Slot2Menu.Slot2FavoritesMenu1.Slot2WaterTemperature -> Flow.ButtonTriggeredMenu.Menu.MainSubMenu.SettingsSubMenu.FavoritesSubMenu.Slot2Menu.Slot2FavoritesMenu1.Slot2Battery [commented out] : UP
                  (junction 8815) -> Flow.ButtonTriggeredMenu.Menu.MainSubMenu.SettingsSubMenu.FavoritesSubMenu.Slot2Menu.Slot2FavoritesMenu1.Slot2WaterTemperature [commented out] : [favSlot2Sel ==eStateName.FAVORITES_WATER_TEMPERATURE ]
                  (junction 8815) -> (junction 8816) [commented out]
                  Flow.ButtonTriggeredMenu.Menu.MainSubMenu.SettingsSubMenu.FavoritesSubMenu.Slot2Menu.Slot2FavoritesMenu1.Slot2WaterTemperature -> Flow.ButtonTriggeredMenu.Menu.MainSubMenu.SettingsSubMenu.FavoritesSubMenu.Slot2Menu.Slot2FavoritesMenu1.Slot2Odometer [commented out] : DOWN
                  Flow.ButtonTriggeredMenu.Menu.MainSubMenu.SettingsSubMenu.FavoritesSubMenu.Slot2Menu.Slot2FavoritesMenu1.Slot2Odometer -> Flow.ButtonTriggeredMenu.Menu.MainSubMenu.SettingsSubMenu.FavoritesSubMenu.Slot2Menu.Slot2FavoritesMenu1.Slot2WaterTemperature [commented out] : UP
                  (junction 8816) -> Flow.ButtonTriggeredMenu.Menu.MainSubMenu.SettingsSubMenu.FavoritesSubMenu.Slot2Menu.Slot2FavoritesMenu1.Slot2Odometer [commented out] : [favSlot2Sel ==eStateName.FAVORITES_ODOMETER ]
                  (junction 8816) -> (junction 8819) [commented out]
                  Flow.ButtonTriggeredMenu.Menu.MainSubMenu.SettingsSubMenu.FavoritesSubMenu.Slot2Menu.Slot2FavoritesMenu1.Slot2Odometer -> Flow.ButtonTriggeredMenu.Menu.MainSubMenu.SettingsSubMenu.FavoritesSubMenu.Slot2Menu.Slot2FavoritesMenu1.Slot2Connectivity [commented out] : DOWN
                  Flow.ButtonTriggeredMenu.Menu.MainSubMenu.SettingsSubMenu.FavoritesSubMenu.Slot2Menu.Slot2FavoritesMenu1.Slot2Connectivity -> Flow.ButtonTriggeredMenu.Menu.MainSubMenu.SettingsSubMenu.FavoritesSubMenu.Slot2Menu.Slot2FavoritesMenu1.Slot2Odometer [commented out] : UP
                  Flow.ButtonTriggeredMenu.Menu.MainSubMenu.SettingsSubMenu.FavoritesSubMenu.Slot2Menu.Slot2FavoritesMenu1.Slot2Connectivity -> (junction 8817) [commented out] : DOWN
                  (junction 8818) -> Flow.ButtonTriggeredMenu.Menu.MainSubMenu.SettingsSubMenu.FavoritesSubMenu.Slot2Menu.Slot2FavoritesMenu1.Slot2Connectivity [commented out]
                  (junction 8819) -> Flow.ButtonTriggeredMenu.Menu.MainSubMenu.SettingsSubMenu.FavoritesSubMenu.Slot2Menu.Slot2FavoritesMenu1.Slot2Connectivity [commented out]
                6514 -> Slot2FavoritesMenu
                Slot2FavoritesMenu -> 6515 [order 1] : EXIT
                (junction 6513) -> 6515 : {favSlot2Updater(currentState);}
                Slot2FavoritesMenu -> (junction 6513) [order 2] : SET
              state Slot3Menu
                (history)
                state Slot3FavoritesMenu
                  (history)
                  state Slot3TripDistance
                    en:
                    currentState = eStateName.FAVORITES_TRIP_DISTANCE_1;
                  state Slot3AverageSpeed
                    en:
                    currentState = eStateName.FAVORITES_AVERAGE_SPEED_1;
                  state Slot3AverageFuelCons
                    en:
                    currentState = eStateName.FAVORITES_AVERAGE_FUEL_CONS_1;
                  state Slot3TripTime
                    en:
                    currentState = eStateName.FAVORITES_TRIP_TIME_1;
                  state Slot3AverageTripDistance
                    en:
                    currentState = eStateName.FAVORITES_TRIP_DISTANCE_2;
                  state Slot3AverageSpeed1
                    en:
                    currentState = eStateName.FAVORITES_AVERAGE_SPEED_2;
                  state Slot3AverageFuelCons1
                    en:
                    currentState = eStateName.FAVORITES_AVERAGE_FUEL_CONS_2;
                  state Slot3TripTime2
                    en:
                    currentState = eStateName.FAVORITES_TRIP_TIME_2;
                  state Slot3FuelRange
                    en:
                    currentState = eStateName.FAVORITES_FUEL_RANGE;
                  state Slot3Date
                    en:
                    currentState = eStateName.FAVORITES_DATE;
                  state Slot3Battery
                    en:
                    currentState = eStateName.FAVORITES_BATTERY;
                  state Slot3WaterTemperature
                    en:
                    currentState = eStateName.FAVORITES_WATER_TEMPERATURE;
                  state Slot3Odometer
                    en:
                    currentState = eStateName.FAVORITES_ODOMETER;
                  state Slot3Connectivity
                    en:
                    currentState = eStateName.FAVORITES_CONNECTIVITY;
                  (default) -> (junction 6612)
                  (junction 6612) -> Flow.ButtonTriggeredMenu.Menu.MainSubMenu.SettingsSubMenu.FavoritesSubMenu.Slot3Menu.Slot3FavoritesMenu.Slot3TripDistance
                  Flow.ButtonTriggeredMenu.Menu.MainSubMenu.SettingsSubMenu.FavoritesSubMenu.Slot3Menu.Slot3FavoritesMenu.Slot3TripDistance -> (junction 6613) [order 2] : UP
                  (junction 6613) -> (junction 6615)
                  (junction 6614) -> (junction 6612)
                  Flow.ButtonTriggeredMenu.Menu.MainSubMenu.SettingsSubMenu.FavoritesSubMenu.Slot3Menu.Slot3FavoritesMenu.Slot3TripDistance -> Flow.ButtonTriggeredMenu.Menu.MainSubMenu.SettingsSubMenu.FavoritesSubMenu.Slot3Menu.Slot3FavoritesMenu.Slot3AverageSpeed [order 1] : DOWN
                  Flow.ButtonTriggeredMenu.Menu.MainSubMenu.SettingsSubMenu.FavoritesSubMenu.Slot3Menu.Slot3FavoritesMenu.Slot3AverageSpeed -> Flow.ButtonTriggeredMenu.Menu.MainSubMenu.SettingsSubMenu.FavoritesSubMenu.Slot3Menu.Slot3FavoritesMenu.Slot3TripDistance [order 1] : UP
                  Flow.ButtonTriggeredMenu.Menu.MainSubMenu.SettingsSubMenu.FavoritesSubMenu.Slot3Menu.Slot3FavoritesMenu.Slot3AverageSpeed -> Flow.ButtonTriggeredMenu.Menu.MainSubMenu.SettingsSubMenu.FavoritesSubMenu.Slot3Menu.Slot3FavoritesMenu.Slot3AverageFuelCons [order 2] : DOWN
                  Flow.ButtonTriggeredMenu.Menu.MainSubMenu.SettingsSubMenu.FavoritesSubMenu.Slot3Menu.Slot3FavoritesMenu.Slot3AverageFuelCons -> Flow.ButtonTriggeredMenu.Menu.MainSubMenu.SettingsSubMenu.FavoritesSubMenu.Slot3Menu.Slot3FavoritesMenu.Slot3AverageSpeed [order 1] : UP
                  Flow.ButtonTriggeredMenu.Menu.MainSubMenu.SettingsSubMenu.FavoritesSubMenu.Slot3Menu.Slot3FavoritesMenu.Slot3AverageFuelCons -> Flow.ButtonTriggeredMenu.Menu.MainSubMenu.SettingsSubMenu.FavoritesSubMenu.Slot3Menu.Slot3FavoritesMenu.Slot3TripTime [order 2] : DOWN
                  Flow.ButtonTriggeredMenu.Menu.MainSubMenu.SettingsSubMenu.FavoritesSubMenu.Slot3Menu.Slot3FavoritesMenu.Slot3TripTime -> Flow.ButtonTriggeredMenu.Menu.MainSubMenu.SettingsSubMenu.FavoritesSubMenu.Slot3Menu.Slot3FavoritesMenu.Slot3AverageFuelCons [order 1] : UP
                  Flow.ButtonTriggeredMenu.Menu.MainSubMenu.SettingsSubMenu.FavoritesSubMenu.Slot3Menu.Slot3FavoritesMenu.Slot3TripTime -> Flow.ButtonTriggeredMenu.Menu.MainSubMenu.SettingsSubMenu.FavoritesSubMenu.Slot3Menu.Slot3FavoritesMenu.Slot3AverageTripDistance [order 2] : DOWN
                  Flow.ButtonTriggeredMenu.Menu.MainSubMenu.SettingsSubMenu.FavoritesSubMenu.Slot3Menu.Slot3FavoritesMenu.Slot3AverageTripDistance -> Flow.ButtonTriggeredMenu.Menu.MainSubMenu.SettingsSubMenu.FavoritesSubMenu.Slot3Menu.Slot3FavoritesMenu.Slot3TripTime [order 1] : UP
                  Flow.ButtonTriggeredMenu.Menu.MainSubMenu.SettingsSubMenu.FavoritesSubMenu.Slot3Menu.Slot3FavoritesMenu.Slot3AverageTripDistance -> Flow.ButtonTriggeredMenu.Menu.MainSubMenu.SettingsSubMenu.FavoritesSubMenu.Slot3Menu.Slot3FavoritesMenu.Slot3AverageSpeed1 [order 2] : DOWN
                  Flow.ButtonTriggeredMenu.Menu.MainSubMenu.SettingsSubMenu.FavoritesSubMenu.Slot3Menu.Slot3FavoritesMenu.Slot3AverageSpeed1 -> Flow.ButtonTriggeredMenu.Menu.MainSubMenu.SettingsSubMenu.FavoritesSubMenu.Slot3Menu.Slot3FavoritesMenu.Slot3AverageTripDistance [order 1] : UP
                  Flow.ButtonTriggeredMenu.Menu.MainSubMenu.SettingsSubMenu.FavoritesSubMenu.Slot3Menu.Slot3FavoritesMenu.Slot3AverageSpeed1 -> Flow.ButtonTriggeredMenu.Menu.MainSubMenu.SettingsSubMenu.FavoritesSubMenu.Slot3Menu.Slot3FavoritesMenu.Slot3AverageFuelCons1 [order 2] : DOWN
                  Flow.ButtonTriggeredMenu.Menu.MainSubMenu.SettingsSubMenu.FavoritesSubMenu.Slot3Menu.Slot3FavoritesMenu.Slot3AverageFuelCons1 -> Flow.ButtonTriggeredMenu.Menu.MainSubMenu.SettingsSubMenu.FavoritesSubMenu.Slot3Menu.Slot3FavoritesMenu.Slot3AverageSpeed1 [order 1] : UP
                  Flow.ButtonTriggeredMenu.Menu.MainSubMenu.SettingsSubMenu.FavoritesSubMenu.Slot3Menu.Slot3FavoritesMenu.Slot3AverageFuelCons1 -> Flow.ButtonTriggeredMenu.Menu.MainSubMenu.SettingsSubMenu.FavoritesSubMenu.Slot3Menu.Slot3FavoritesMenu.Slot3TripTime2 [order 2] : DOWN
                  Flow.ButtonTriggeredMenu.Menu.MainSubMenu.SettingsSubMenu.FavoritesSubMenu.Slot3Menu.Slot3FavoritesMenu.Slot3TripTime2 -> Flow.ButtonTriggeredMenu.Menu.MainSubMenu.SettingsSubMenu.FavoritesSubMenu.Slot3Menu.Slot3FavoritesMenu.Slot3AverageFuelCons1 [order 1] : UP
                  Flow.ButtonTriggeredMenu.Menu.MainSubMenu.SettingsSubMenu.FavoritesSubMenu.Slot3Menu.Slot3FavoritesMenu.Slot3TripTime2 -> Flow.ButtonTriggeredMenu.Menu.MainSubMenu.SettingsSubMenu.FavoritesSubMenu.Slot3Menu.Slot3FavoritesMenu.Slot3FuelRange [order 2] : DOWN
                  Flow.ButtonTriggeredMenu.Menu.MainSubMenu.SettingsSubMenu.FavoritesSubMenu.Slot3Menu.Slot3FavoritesMenu.Slot3FuelRange -> Flow.ButtonTriggeredMenu.Menu.MainSubMenu.SettingsSubMenu.FavoritesSubMenu.Slot3Menu.Slot3FavoritesMenu.Slot3TripTime2 [order 1] : UP
                  Flow.ButtonTriggeredMenu.Menu.MainSubMenu.SettingsSubMenu.FavoritesSubMenu.Slot3Menu.Slot3FavoritesMenu.Slot3FuelRange -> Flow.ButtonTriggeredMenu.Menu.MainSubMenu.SettingsSubMenu.FavoritesSubMenu.Slot3Menu.Slot3FavoritesMenu.Slot3Date [order 2] : DOWN
                  Flow.ButtonTriggeredMenu.Menu.MainSubMenu.SettingsSubMenu.FavoritesSubMenu.Slot3Menu.Slot3FavoritesMenu.Slot3Date -> Flow.ButtonTriggeredMenu.Menu.MainSubMenu.SettingsSubMenu.FavoritesSubMenu.Slot3Menu.Slot3FavoritesMenu.Slot3FuelRange [order 1] : UP
                  Flow.ButtonTriggeredMenu.Menu.MainSubMenu.SettingsSubMenu.FavoritesSubMenu.Slot3Menu.Slot3FavoritesMenu.Slot3Date -> Flow.ButtonTriggeredMenu.Menu.MainSubMenu.SettingsSubMenu.FavoritesSubMenu.Slot3Menu.Slot3FavoritesMenu.Slot3Battery [order 2] : DOWN
                  Flow.ButtonTriggeredMenu.Menu.MainSubMenu.SettingsSubMenu.FavoritesSubMenu.Slot3Menu.Slot3FavoritesMenu.Slot3Battery -> Flow.ButtonTriggeredMenu.Menu.MainSubMenu.SettingsSubMenu.FavoritesSubMenu.Slot3Menu.Slot3FavoritesMenu.Slot3Date [order 1] : UP
                  Flow.ButtonTriggeredMenu.Menu.MainSubMenu.SettingsSubMenu.FavoritesSubMenu.Slot3Menu.Slot3FavoritesMenu.Slot3Battery -> Flow.ButtonTriggeredMenu.Menu.MainSubMenu.SettingsSubMenu.FavoritesSubMenu.Slot3Menu.Slot3FavoritesMenu.Slot3WaterTemperature [order 2] : DOWN
                  Flow.ButtonTriggeredMenu.Menu.MainSubMenu.SettingsSubMenu.FavoritesSubMenu.Slot3Menu.Slot3FavoritesMenu.Slot3WaterTemperature -> Flow.ButtonTriggeredMenu.Menu.MainSubMenu.SettingsSubMenu.FavoritesSubMenu.Slot3Menu.Slot3FavoritesMenu.Slot3Battery [order 1] : UP
                  Flow.ButtonTriggeredMenu.Menu.MainSubMenu.SettingsSubMenu.FavoritesSubMenu.Slot3Menu.Slot3FavoritesMenu.Slot3WaterTemperature -> Flow.ButtonTriggeredMenu.Menu.MainSubMenu.SettingsSubMenu.FavoritesSubMenu.Slot3Menu.Slot3FavoritesMenu.Slot3Odometer [order 2] : DOWN
                  Flow.ButtonTriggeredMenu.Menu.MainSubMenu.SettingsSubMenu.FavoritesSubMenu.Slot3Menu.Slot3FavoritesMenu.Slot3Odometer -> Flow.ButtonTriggeredMenu.Menu.MainSubMenu.SettingsSubMenu.FavoritesSubMenu.Slot3Menu.Slot3FavoritesMenu.Slot3WaterTemperature [order 1] : UP
                  Flow.ButtonTriggeredMenu.Menu.MainSubMenu.SettingsSubMenu.FavoritesSubMenu.Slot3Menu.Slot3FavoritesMenu.Slot3Odometer -> Flow.ButtonTriggeredMenu.Menu.MainSubMenu.SettingsSubMenu.FavoritesSubMenu.Slot3Menu.Slot3FavoritesMenu.Slot3Connectivity [order 2] : DOWN